simulink model "large_model"  (Simulink 8.0)
settings: Solver=ode45  StartTime=0.0  StopTime=10.0  RelTol=1e-3
(part 1 of 21)
settings Model: Creator=eng006  LastModifiedBy=rstarr
settings DataIOCC: SignalLoggingSaveFormat=ModelDataLogs
settings DebuggingCC: FcnCallInpInsideContextMsg=UseLocalSettings
settings GRTTargetCC: CombineOutputUpdateFcns=off  GRTInterface=on

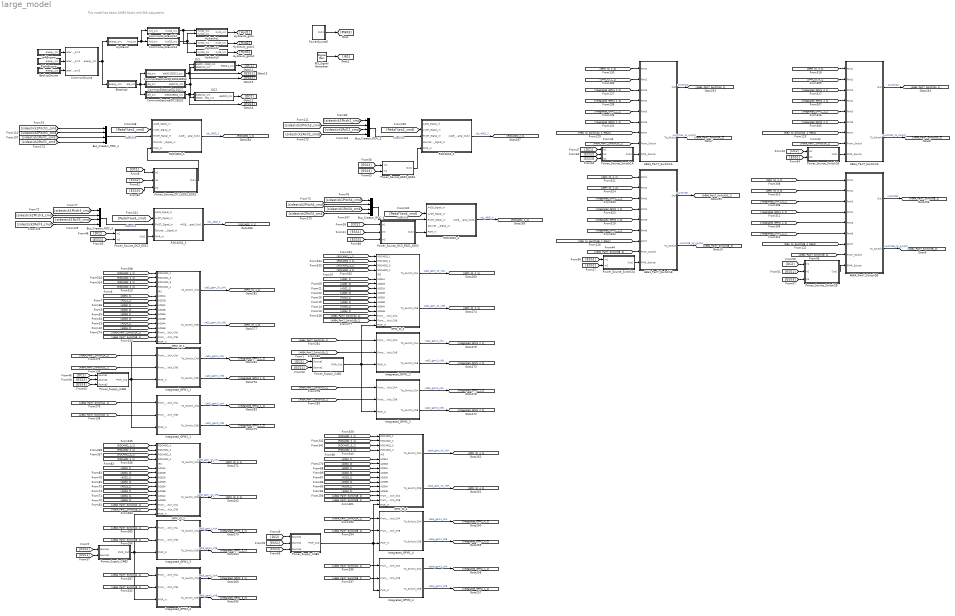
== system large_model ==
  note: This model has about 10959 blocks and 593 subsystems
  A664_Part7_Switch1A : Subsystem
  A664_Part7_Switch1B : Subsystem
  A664_Part7_Switch2A : Subsystem
  A664_Part7_Switch2B : Subsystem
  BackupSource : Subsystem
  Bus_Creator_RDC_{1..4} (4 alike) : BusCreator
  CommonElectricalDC1DC2 : Subsystem
  CommonElectricalDC1ESS1ESS3 : Subsystem
  CommonElectricalDC2ESS2 : Subsystem
  CommonHydraulic12 : Subsystem
  CommonHydraulic13 : Subsystem
  CommonSource : Subsystem
  DC1 : Subsystem
  DC2 : Subsystem
  Electrical : Subsystem
  GPM_IO_1 : Subsystem
  GPM_IO_2 : Subsystem
  GPM_IO_3 : Subsystem
  GPM_IO_4 : Subsystem
  Hydraulic : Subsystem
  Hydraulic1 : Subsystem
  Hydraulic2 : Subsystem
  Hydraulic3 : Subsystem
  IRS_Signal Generator : SignalGenerator
  Integrated_GPM1_1 : Subsystem
  Integrated_GPM1_2 : Subsystem
  Integrated_GPM1_3 : Subsystem
  Integrated_GPM1_4 : Subsystem
  Integrated_GPM2_1 : Subsystem
  Integrated_GPM2_2 : Subsystem
  Integrated_GPM2_3 : Subsystem
  Integrated_GPM2_4 : Subsystem
  LeftEngine : Subsystem
  PowerSource5 : Subsystem
  Power_Source_DC1_ESS2_ESS3 : Subsystem
  Power_Source_DC2_ESS1 : Subsystem
  Power_Source_DC2_ESS1_ESS3 : Subsystem
  Power_Source_ESS2_ESS3 : Subsystem
  Power_Source_Switch1A : Subsystem
  Power_Source_Switch1B : Subsystem
  Power_Source_Switch2A : Subsystem
  Power_Source_Switch2B : Subsystem
  Power_Supply_CAB1 : Subsystem
  Power_Supply_CAB2 : Subsystem
  Power_Supply_CAB3 : Subsystem
  Power_Supply_CAB4 : Subsystem
  RDC4002_1 : Subsystem
  RDC4002_2 : Subsystem
  RDC4002_3 : Subsystem
  RDC4002_4 : Subsystem
  RightEngine : Subsystem
  wires:
  Power_Supply_CAB1/1(PWR_Out) -> Integrated_GPM1_1/3(PWR_In), Integrated_GPM2_1/3(PWR_In), GPM_IO_1/16(PWR_In)
  A664_Part7_Switch2A/1(Out) -> [A664_Part7_Switch2A_1]  "switch2A"
  Power_Supply_CAB2/1(PWR_Out) -> GPM_IO_2/16(PWR_In), Integrated_GPM2_2/3(PWR_In), Integrated_GPM1_2/3(PWR_In)
  PowerSource5/1(Out1) -> [PWR3]
  IRS_Signal Generator/1 -> [IRS]
  Bus_Creator_RDC_1/1 -> RDC4002_1/3(RVDT_Signal_In)  "sidesticks"
  Bus_Creator_RDC_2/1 -> RDC4002_2/3(RVDT_Signal_In)  "sidesticks"
  Bus_Creator_RDC_3/1 -> RDC4002_3/3(RVDT_Signal_In)  "sidesticks"
  Bus_Creator_RDC_4/1 -> RDC4002_4/3(RVDT_Signal_In)  "sidesticks"
  Power_Supply_CAB3/1(PWR_Out) -> GPM_IO_3/16(PWR_In), Integrated_GPM1_3/3(PWR_In), Integrated_GPM2_3/3(PWR_In)
  A664_Part7_Switch1B/1(Out) -> [A664_Part7_Switch1B_1]  "switch1B"
  A664_Part7_Switch2B/1(Out) -> [A664_Part7_Switch2B_1]  "switch2B"
  Power_Supply_CAB4/1(PWR_Out) -> Integrated_GPM2_4/3(PWR_In), Integrated_GPM1_4/3(PWR_In), GPM_IO_4/16(PWR_In)
  [DC1] -> Power_Supply_CAB1/1(Source1)
  [DC2] -> Power_Supply_CAB2/1(Source1)
  [DC2] -> Power_Supply_CAB4/1(Source1)
  [ESS1] -> Power_Supply_CAB4/2(Source2)
  [ESS1] -> Power_Supply_CAB2/2(Source2)
  [ESS1] -> Power_Supply_CAB3/2(Source2)
  [ESS2] -> Power_Supply_CAB3/1(Source1)
  [ESS2] -> Power_Supply_CAB1/2(Source2)
  [DC2] -> Power_Source_DC2_ESS1_ESS3/1(In1)
  [ESS1] -> Power_Source_DC2_ESS1_ESS3/2(In2)
  [ESS3] -> Power_Source_DC2_ESS1_ESS3/3(In3)
  Power_Source_DC2_ESS1_ESS3/1(Out1) -> RDC4002_4/5(PWR_In)
  [DC2] -> Power_Source_DC2_ESS1/1(In1)
  [ESS1] -> Power_Source_DC2_ESS1/2(In2)
  Power_Source_DC2_ESS1/1(Out1) -> RDC4002_3/5(PWR_In)
  [ESS2] -> Power_Source_ESS2_ESS3/1(In1)
  [ESS3] -> Power_Source_ESS2_ESS3/2(In2)
  Power_Source_ESS2_ESS3/1(Out1) -> RDC4002_2/5(PWR_In)
  [DC1] -> Power_Source_DC1_ESS2_ESS3/1(In1)
  [ESS2] -> Power_Source_DC1_ESS2_ESS3/2(In2)
  [ESS3] -> Power_Source_DC1_ESS2_ESS3/3(In3)
  Power_Source_DC1_ESS2_ESS3/1(Out1) -> RDC4002_1/5(PWR_In)
  [DC2] -> Power_Source_Switch2B/1(In1)
  [ESS1] -> Power_Source_Switch2B/2(In2)
  [ESS3] -> Power_Source_Switch2B/3(In3)
  [ESS2] -> Power_Source_Switch2A/2(In2)
  [ESS1] -> Power_Source_Switch2A/1(In1)
  Power_Source_Switch2A/1(Out1) -> A664_Part7_Switch1B/9(PWR_Source)
  [DC2] -> Power_Source_Switch1B/1(In1)
  [ESS1] -> Power_Source_Switch1B/2(In2)
  Power_Source_Switch1B/1(Out1) -> A664_Part7_Switch2A/9(PWR_Source)
  [DC1] -> Power_Source_Switch1A/1(In1)
  [ESS2] -> Power_Source_Switch1A/2(In2)
  [ESS3] -> Power_Source_Switch1A/3(In3)
  Power_Source_Switch1A/1(Out1) -> A664_Part7_Switch1A/9(PWR_Source)
  [ESS3] -> Power_Supply_CAB4/3(Source3)
  [ESS3] -> Power_Supply_CAB1/3(Source3)
  [sidestick1Pitch1_cmd] -> Bus_Creator_RDC_1/1
  [sidestick1Pitch2_cmd] -> Bus_Creator_RDC_2/1
  [sidestick1Pitch3_cmd] -> Bus_Creator_RDC_3/1
  [sidestick1Pitch4_cmd] -> Bus_Creator_RDC_4/1
  [sidestick2Pitch1_cmd] -> Bus_Creator_RDC_1/2
  [PedalYaw1_cmd] -> RDC4002_1/2(LVDT_Signal_In)
  [sidestick2Pitch2_cmd] -> Bus_Creator_RDC_2/2
  [PedalYaw2_cmd] -> RDC4002_2/2(LVDT_Signal_In)
  [PedalYaw3_cmd] -> RDC4002_3/2(LVDT_Signal_In)
  [PedalYaw4_cmd] -> RDC4002_4/2(LVDT_Signal_In)
  [sidestick2Pitch3_cmd] -> Bus_Creator_RDC_3/2
  [sidestick2Pitch4_cmd] -> Bus_Creator_RDC_4/2
  [sidestick1Roll1_cmd] -> Bus_Creator_RDC_1/3
  [sidestick2Roll1_cmd] -> Bus_Creator_RDC_1/4
  [sidestick1Roll2_cmd] -> Bus_Creator_RDC_2/3
  [sidestick2Roll2_cmd] -> Bus_Creator_RDC_2/4
  [sidestick1Roll3_cmd] -> Bus_Creator_RDC_3/3
  [sidestick2Roll3_cmd] -> Bus_Creator_RDC_3/4
  [sidestick1Roll4_cmd] -> Bus_Creator_RDC_4/3
  [sidestick2Roll4_cmd] -> Bus_Creator_RDC_4/4
  [ADS2_2] -> GPM_IO_4/9(ADS2B)
  [ADS3_2] -> GPM_IO_4/11(ADS3B)
  [ADS4_2] -> GPM_IO_4/13(ADS4B)
  A664_Part7_Switch1A/2(To_Switch) -> [A664_Part7_Switch1A_2]  "switch1A_to_switch"
  [A664_Part7_Switch1A_2] -> A664_Part7_Switch2A/8(From_Switch)
  A664_Part7_Switch2A/2(To_Switch) -> [A664_Part7_Switch2A_2]  "switch2A_to_switch"
  [A664_Part7_Switch2A_2] -> A664_Part7_Switch1A/8(From_Switch)
  A664_Part7_Switch1A/1(Out) -> [A664_Part7_Switch1A_1]  "switch1A"
  A664_Part7_Switch2B/2(To_Switch) -> [A664_Part7_Switch2B_2]  "switch2B_to_switch"
  [A664_Part7_Switch2B_2] -> A664_Part7_Switch1B/8(From_Switch)
  A664_Part7_Switch1B/2(To_Switch) -> [A664_Part7_Switch1B_2]  "switch1B_to_switch"
  [A664_Part7_Switch1B_2] -> A664_Part7_Switch2B/8(From_Switch)
  [A664_Part7_Switch1A_1] -> GPM_IO_1/14(From_Switch_ChA)
  [A664_Part7_Switch1A_1] -> Integrated_GPM1_1/1(From_Switch_ChA)
  [A664_Part7_Switch1A_1] -> Integrated_GPM2_1/1(From_Switch_ChA)
  [A664_Part7_Switch1B_1] -> GPM_IO_2/15(From_Switch_ChB)
  [A664_Part7_Switch1B_1] -> Integrated_GPM2_1/2(From_Switch_ChB)
  [A664_Part7_Switch1B_1] -> Integrated_GPM1_1/2(From_Switch_ChB)
  [A664_Part7_Switch1B_1] -> GPM_IO_1/15(From_Switch_ChB)
  [A664_Part7_Switch1A_1] -> GPM_IO_2/14(From_Switch_ChA)
  [A664_Part7_Switch1A_1] -> Integrated_GPM1_2/1(From_Switch_ChA)
  [A664_Part7_Switch1B_1] -> Integrated_GPM2_2/2(From_Switch_ChB)
  [A664_Part7_Switch1B_1] -> Integrated_GPM1_2/2(From_Switch_ChB)
  [A664_Part7_Switch1A_1] -> Integrated_GPM2_2/1(From_Switch_ChA)
  [A664_Part7_Switch2A_1] -> Integrated_GPM1_3/1(From_Switch_ChA)
  [A664_Part7_Switch2A_1] -> Integrated_GPM2_3/1(From_Switch_ChA)
  [A664_Part7_Switch1B_1] -> GPM_IO_4/14(From_Switch_ChA)
  [A664_Part7_Switch2A_1] -> Integrated_GPM1_4/1(From_Switch_ChA)
  [A664_Part7_Switch2A_1] -> Integrated_GPM2_4/1(From_Switch_ChA)
  [A664_Part7_Switch2B_1] -> Integrated_GPM1_3/2(From_Switch_ChB)
  [A664_Part7_Switch2B_1] -> Integrated_GPM2_3/2(From_Switch_ChB)
  [A664_Part7_Switch2B_1] -> GPM_IO_4/15(From_Switch_ChB)
  [A664_Part7_Switch2B_1] -> Integrated_GPM1_4/2(From_Switch_ChB)
  [A664_Part7_Switch2B_1] -> Integrated_GPM2_4/2(From_Switch_ChB)
  Integrated_GPM2_4/2(To_Switch_ChB) -> [Integrated_GPM2_4_2]  "cab4_gpm2_chB"
  [Integrated_GPM2_4_2] -> A664_Part7_Switch2B/5(Port5)
  Integrated_GPM2_4/1(To_Switch_ChA) -> [Integrated_GPM2_4_1]  "cab4_gpm2_chA"
  Integrated_GPM1_4/2(To_Switch_ChB) -> [Integrated_GPM1_4_2]  "cab4_gpm1_chB"
  [Integrated_GPM1_4_2] -> A664_Part7_Switch2B/3(Port3)
  Integrated_GPM1_4/1(To_Switch_ChA) -> [Integrated_GPM1_4_1]  "cab4_gpm1_chA"
  GPM_IO_4/2(To_Switch_ChB) -> [GPM_IO_4_2]  "cab4_gpm_IO_chB"
  [GPM_IO_4_2] -> A664_Part7_Switch2B/1(Port1)
  GPM_IO_4/1(To_Switch_ChA) -> [GPM_IO_4_1]  "cab4_gpm_IO_chA"
  GPM_IO_3/2(To_Switch_ChB) -> [GPM_IO_3_2]  "cab3_gpm_IO_chB"
  [GPM_IO_3_2] -> A664_Part7_Switch2B/2(Port2)
  Integrated_GPM1_3/2(To_Switch_ChB) -> [Integrated_GPM1_3_2]  "cab3_gpm1_chB"
  [Integrated_GPM1_3_2] -> A664_Part7_Switch2B/4(Port4)
  Integrated_GPM2_3/2(To_Switch_ChB) -> [Integrated_GPM2_3_2]  "cab3_gpm2_chB"
  [Integrated_GPM2_3_2] -> A664_Part7_Switch2B/6(Port6)
  Integrated_GPM2_3/1(To_Switch_ChA) -> [Integrated_GPM2_3_1]  "cab3_gpm2_chA"
  Integrated_GPM1_3/1(To_Switch_ChA) -> [Integrated_GPM1_3_1]  "cab3_gpm1_chA"
  GPM_IO_3/1(To_Switch_ChA) -> [GPM_IO_3_1]  "cab3_gpm_IO_chA"
  Power_Source_Switch2B/1(Out1) -> A664_Part7_Switch2B/9(PWR_Source)
  Integrated_GPM2_2/2(To_Switch_ChB) -> [Integrated_GPM2_2_2]  "cab2_gpm2_chB"
  Integrated_GPM1_2/2(To_Switch_ChB) -> [Integrated_GPM1_2_2]  "cab2_gpm1_chB"
  GPM_IO_2/2(To_Switch_ChB) -> [GPM_IO_2_2]  "cab2_gpm_IO_chB"
  Integrated_GPM2_1/2(To_Switch_ChB) -> [Integrated_GPM2_1_2]  "cab1_gpm2_chB"
  Integrated_GPM1_1/2(To_Switch_ChB) -> [Integrated_GPM1_1_2]  "cab1_gpm1_chB"
  GPM_IO_1/2(To_Switch_ChB) -> [GPM_IO_1_2]  "cab1_gpm_IO_chB"
  Integrated_GPM2_2/1(To_Switch_ChA) -> [Integrated_GPM2_2_1]  "cab2_gpm2_chA"
  Integrated_GPM1_2/1(To_Switch_ChA) -> [Integrated_GPM1_2_1]  "cab2_gpm1_chA"
  [Integrated_GPM1_1_2] -> A664_Part7_Switch1A/4(Port4)
  GPM_IO_2/1(To_Switch_ChA) -> [GPM_IO_2_1]  "cab2_gpm_IO_chA"
  Integrated_GPM1_1/1(To_Switch_ChA) -> [Integrated_GPM1_1_1]  "cab1_gpm1_chA"
  [Integrated_GPM1_2_2] -> A664_Part7_Switch1A/3(Port3)
  GPM_IO_1/1(To_Switch_ChA) -> [GPM_IO_1_1]  "cab1_gpm_IO_chA"
  [GPM_IO_2_2] -> A664_Part7_Switch1A/1(Port1)
  Integrated_GPM2_1/1(To_Switch_ChA) -> [Integrated_GPM2_1_1]  "cab1_gpm2_chA"
  [ADS1_1] -> GPM_IO_1/6(ADS1A)
  [ADS1_1] -> GPM_IO_4/6(ADS1A)
  [ADS1_2] -> GPM_IO_1/7(ADS1B)
  [ADS1_2] -> GPM_IO_4/7(ADS1B)
  [ADS2_1] -> GPM_IO_1/8(ADS2A)
  [ADS2_1] -> GPM_IO_4/8(ADS2A)
  [ADS4_2] -> GPM_IO_1/13(ADS4B)
  [ADS4_1] -> GPM_IO_1/12(ADS4A)
  [ADS3_2] -> GPM_IO_1/11(ADS3B)
  [ADS3_1] -> GPM_IO_1/10(ADS3A)
  [ADS2_2] -> GPM_IO_1/9(ADS2B)
  [ADS1_1] -> GPM_IO_2/6(ADS1A)
  [ADS1_2] -> GPM_IO_2/7(ADS1B)
  [ADS2_1] -> GPM_IO_2/8(ADS2A)
  [ADS2_2] -> GPM_IO_2/9(ADS2B)
  [ADS3_1] -> GPM_IO_2/10(ADS3A)
  [ADS3_2] -> GPM_IO_2/11(ADS3B)
  [ADS4_1] -> GPM_IO_2/12(ADS4A)
  [ADS4_2] -> GPM_IO_2/13(ADS4B)
  [ADS1_1] -> GPM_IO_3/6(ADS1A)
  [ADS1_2] -> GPM_IO_3/7(ADS1B)
  [ADS2_1] -> GPM_IO_3/8(ADS2A)
  [ADS2_2] -> GPM_IO_3/9(ADS2B)
  [ADS3_1] -> GPM_IO_3/10(ADS3A)
  [ADS3_2] -> GPM_IO_3/11(ADS3B)
  [ADS4_1] -> GPM_IO_3/12(ADS4A)
  [ADS4_2] -> GPM_IO_3/13(ADS4B)
  [ADS3_1] -> GPM_IO_4/10(ADS3A)
  [ADS4_1] -> GPM_IO_4/12(ADS4A)
  [A664_Part7_Switch2A_1] -> GPM_IO_3/14(From_Switch_ChA)
  [A664_Part7_Switch2B_1] -> GPM_IO_3/15(From_Switch_ChB)
  RDC4002_1/1(A429_Signal_Out1) -> [RDC4002_1_1]  "rdc_4002_1"
  [RDC4002_1_1] -> GPM_IO_1/1(RDC4002_1)
  RDC4002_4/1(A429_Signal_Out1) -> [RDC4002_4_1]  "rdc_4002_4"
  [RDC4002_4_1] -> GPM_IO_1/4(RDC4002_4)
  RDC4002_3/1(A429_Signal_Out1) -> [RDC4002_3_1]  "rdc_4002_3"
  [RDC4002_3_1] -> GPM_IO_1/3(RDC4002_3)
  RDC4002_2/1(A429_Signal_Out1) -> [RDC4002_2_1]  "rdc_4002_2"
  [RDC4002_2_1] -> GPM_IO_1/2(RDC4002_2)
  [RDC4002_1_1] -> GPM_IO_2/1(RDC4002_1)
  [RDC4002_2_1] -> GPM_IO_2/2(RDC4002_2)
  [RDC4002_3_1] -> GPM_IO_2/3(RDC4002_3)
  [RDC4002_4_1] -> GPM_IO_2/4(RDC4002_4)
  [RDC4002_1_1] -> GPM_IO_3/1(RDC4002_1)
  [RDC4002_2_1] -> GPM_IO_3/2(RDC4002_2)
  [RDC4002_3_1] -> GPM_IO_3/3(RDC4002_3)
  [RDC4002_4_1] -> GPM_IO_3/4(RDC4002_4)
  [RDC4002_1_1] -> GPM_IO_4/1(RDC4002_1)
  [RDC4002_2_1] -> GPM_IO_4/2(RDC4002_2)
  [RDC4002_3_1] -> GPM_IO_4/3(RDC4002_3)
  [RDC4002_4_1] -> GPM_IO_4/4(RDC4002_4)
  [GPM_IO_2_2] -> A664_Part7_Switch1B/1(Port1)
  [GPM_IO_1_2] -> A664_Part7_Switch1B/2(Port2)
  [Integrated_GPM1_2_2] -> A664_Part7_Switch1B/3(Port3)
  [Integrated_GPM1_1_2] -> A664_Part7_Switch1B/4(Port4)
  [Integrated_GPM2_2_2] -> A664_Part7_Switch1B/5(Port5)
  [Integrated_GPM2_1_2] -> A664_Part7_Switch1B/6(Port6)
  [GPM_IO_3_1] -> A664_Part7_Switch2A/1(Port1)
  [GPM_IO_4_1] -> A664_Part7_Switch2A/2(Port2)
  [Integrated_GPM1_3_1] -> A664_Part7_Switch2A/3(Port3)
  [Integrated_GPM1_4_1] -> A664_Part7_Switch2A/4(Port4)
  [Integrated_GPM2_3_1] -> A664_Part7_Switch2A/5(Port5)
  [Integrated_GPM2_4_1] -> A664_Part7_Switch2A/6(Port6)
  [GPM_IO_1_2] -> A664_Part7_Switch1A/2(Port2)
  [Integrated_GPM2_2_2] -> A664_Part7_Switch1A/5(Port5)
  [Integrated_GPM2_1_2] -> A664_Part7_Switch1A/6(Port6)
  CommonSource/1(energy_src) -> Electrical/1(energy_src), Hydraulic/1(energy_src)
  BackupSource/1(energy_src) -> CommonSource/3(energy_src3)
  RightEngine/1(energy_src) -> CommonSource/2(energy_src2)
  LeftEngine/1(energy_src) -> CommonSource/1(energy_src1)
  CommonHydraulic13/1(hyd13_src) -> Hydraulic3/1(hyd13_src), Hydraulic1/2(hyd13_src)
  CommonHydraulic12/1(hyd12_src) -> Hydraulic2/1(hyd12_src), Hydraulic1/1(hyd12_src)
  Hydraulic/1(hyd_src) -> CommonHydraulic12/1(hyd_src), CommonHydraulic13/1(hyd_src)
  CommonElectricalDC1DC2/1(eleDC12_src) -> DC1/2(eleDC12_src), DC2/2(eleDC2ESS2_src)
  DC2/1(eleDC2_src) -> [DC2]
  DC1/1(eleDC1_src) -> [DC1]
  CommonElectricalDC2ESS2/1(eleDC2ESS2_src) -> [ESS3], DC2/1(eleDC12_src)
  CommonElectricalDC1ESS1ESS3/1(eleDC1ESS13_src) -> [ESS2], [ESS1], DC1/1(eleDC1ESS13_src)
  Electrical/1(ele_src) -> CommonElectricalDC1ESS1ESS3/1(ele_src), CommonElectricalDC2ESS2/1(ele_src), CommonElectricalDC1DC2/1(ele_src)
  Hydraulic1/1(hyd1_src) -> [Hyd1]
  Hydraulic2/1(hyd2_src) -> [Hyd2]
  Hydraulic3/1(hyd3_src) -> [Hyd3]
  [REU_to_Switch2B_1_REUs] -> A664_Part7_Switch2B/7(Port7)
  [REU_to_Switch2A_1_REUs] -> A664_Part7_Switch2A/7(Port7)
  [REU_to_Switch1B_1_REUs] -> A664_Part7_Switch1B/7(Port7)
  [REU_to_Switch1A_1_REUs] -> A664_Part7_Switch1A/7(Port7)

== system large_model/A664_Part7_Switch1A ==
  note: Atenção! O sinal de From_Switch não deve ser propagado para a saída To_Switch.
      | Isto é feito para evitar loops entre switches.
  Port1 : Inport
  Port2 : Inport  Port=2
  Port3 : Inport  Port=3
  Port4 : Inport  Port=4
  Port5 : Inport  Port=5
  Port6 : Inport  Port=6
  Port7 : Inport  Port=7
  From_Switch : Inport  Port=8
  PWR_Source : Inport  Port=9
  Bus Direct : BusCreator  Inputs=8
  Bus to Switch : BusCreator  Inputs=7
  Switch Hardware : Subsystem
  Out : Outport
  To_Switch : Outport  Port=2
  wires:
  Bus Direct/1 -> Switch Hardware/1(In1)
  PWR_Source/1 -> Switch Hardware/3(PWR)
  Switch Hardware/1(Out1) -> Out/1
  Switch Hardware/2(Out2) -> To_Switch/1
  Bus to Switch/1 -> Switch Hardware/2(In2)
  Port1/1 -> Bus Direct/1, Bus to Switch/1
  Port2/1 -> Bus Direct/2, Bus to Switch/2
  Port3/1 -> Bus Direct/3, Bus to Switch/3
  Port4/1 -> Bus Direct/4, Bus to Switch/4
  Port5/1 -> Bus Direct/5, Bus to Switch/5
  Port6/1 -> Bus Direct/6, Bus to Switch/6
  Port7/1 -> Bus Direct/7, Bus to Switch/7
  From_Switch/1 -> Bus Direct/8

== system large_model/A664_Part7_Switch1B ==
  note: Atenção! O sinal de From_Switch não deve ser propagado para a saída To_Switch.
      | Isto é feito para evitar loops entre switches.
  Port1 : Inport
  Port2 : Inport  Port=2
  Port3 : Inport  Port=3
  Port4 : Inport  Port=4
  Port5 : Inport  Port=5
  Port6 : Inport  Port=6
  Port7 : Inport  Port=7
  From_Switch : Inport  Port=8
  PWR_Source : Inport  Port=9
  Bus Direct : BusCreator  Inputs=8
  Bus to Switch : BusCreator  Inputs=7
  Switch Hardware : Subsystem
  Out : Outport
  To_Switch : Outport  Port=2
  wires:
  Bus Direct/1 -> Switch Hardware/1(In1)
  PWR_Source/1 -> Switch Hardware/3(PWR)
  Switch Hardware/1(Out1) -> Out/1
  Switch Hardware/2(Out2) -> To_Switch/1
  Bus to Switch/1 -> Switch Hardware/2(In2)
  Port1/1 -> Bus Direct/1, Bus to Switch/1
  Port2/1 -> Bus Direct/2, Bus to Switch/2
  Port3/1 -> Bus Direct/3, Bus to Switch/3
  Port4/1 -> Bus Direct/4, Bus to Switch/4
  Port5/1 -> Bus Direct/5, Bus to Switch/5
  Port6/1 -> Bus Direct/6, Bus to Switch/6
  Port7/1 -> Bus Direct/7, Bus to Switch/7
  From_Switch/1 -> Bus Direct/8

== system large_model/A664_Part7_Switch2A ==
  note: Atenção! O sinal de From_Switch não deve ser propagado para a saída To_Switch.
      | Isto é feito para evitar loops entre switches.
  Port1 : Inport
  Port2 : Inport  Port=2
  Port3 : Inport  Port=3
  Port4 : Inport  Port=4
  Port5 : Inport  Port=5
  Port6 : Inport  Port=6
  Port7 : Inport  Port=7
  From_Switch : Inport  Port=8
  PWR_Source : Inport  Port=9
  Bus Direct : BusCreator  Inputs=8
  Bus to Switch : BusCreator  Inputs=7
  Switch Hardware : Subsystem
  Out : Outport
  To_Switch : Outport  Port=2
  wires:
  Bus Direct/1 -> Switch Hardware/1(In1)
  PWR_Source/1 -> Switch Hardware/3(PWR)
  Switch Hardware/1(Out1) -> Out/1
  Switch Hardware/2(Out2) -> To_Switch/1
  Bus to Switch/1 -> Switch Hardware/2(In2)
  Port1/1 -> Bus Direct/1, Bus to Switch/1
  Port2/1 -> Bus Direct/2, Bus to Switch/2
  Port3/1 -> Bus Direct/3, Bus to Switch/3
  Port4/1 -> Bus Direct/4, Bus to Switch/4
  Port5/1 -> Bus Direct/5, Bus to Switch/5
  Port6/1 -> Bus Direct/6, Bus to Switch/6
  Port7/1 -> Bus Direct/7, Bus to Switch/7
  From_Switch/1 -> Bus Direct/8

== system large_model/A664_Part7_Switch2B ==
  note: Atenção! O sinal de From_Switch não deve ser propagado para a saída To_Switch.
      | Isto é feito para evitar loops entre switches.
  Port1 : Inport
  Port2 : Inport  Port=2
  Port3 : Inport  Port=3
  Port4 : Inport  Port=4
  Port5 : Inport  Port=5
  Port6 : Inport  Port=6
  Port7 : Inport  Port=7
  From_Switch : Inport  Port=8
  PWR_Source : Inport  Port=9
  Bus Direct : BusCreator  Inputs=8
  Bus to Switch : BusCreator  Inputs=7
  Switch Hardware : Subsystem
  Out : Outport
  To_Switch : Outport  Port=2
  wires:
  Bus Direct/1 -> Switch Hardware/1(In1)
  PWR_Source/1 -> Switch Hardware/3(PWR)
  Switch Hardware/1(Out1) -> Out/1
  Switch Hardware/2(Out2) -> To_Switch/1
  Bus to Switch/1 -> Switch Hardware/2(In2)
  Port1/1 -> Bus Direct/1, Bus to Switch/1
  Port2/1 -> Bus Direct/2, Bus to Switch/2
  Port3/1 -> Bus Direct/3, Bus to Switch/3
  Port4/1 -> Bus Direct/4, Bus to Switch/4
  Port5/1 -> Bus Direct/5, Bus to Switch/5
  Port6/1 -> Bus Direct/6, Bus to Switch/6
  Port7/1 -> Bus Direct/7, Bus to Switch/7
  From_Switch/1 -> Bus Direct/8

== system large_model/BackupSource ==
  energy_src : Outport

== system large_model/CommonElectricalDC1DC2 ==
  ele_src : Inport
  eleDC12_src : Outport

== system large_model/CommonElectricalDC1ESS1ESS3 ==
  ele_src : Inport
  eleDC1ESS13_src : Outport

== system large_model/CommonElectricalDC2ESS2 ==
  ele_src : Inport
  eleDC2ESS2_src : Outport

== system large_model/CommonHydraulic12 ==
  hyd_src : Inport
  hyd12_src : Outport

== system large_model/CommonHydraulic13 ==
  hyd_src : Inport
  hyd13_src : Outport

== system large_model/CommonSource ==
  energy_src1 : Inport
  energy_src2 : Inport  Port=2
  energy_src3 : Inport  Port=3
  energy_src : Outport

== system large_model/DC1 ==
  eleDC1ESS13_src : Inport
  eleDC12_src : Inport  Port=2
  eleDC1_src : Outport

== system large_model/DC2 ==
  eleDC12_src : Inport
  eleDC2ESS2_src : Inport  Port=2
  eleDC2_src : Outport

== system large_model/Electrical ==
  energy_src : Inport
  ele_src : Outport

== system large_model/GPM_IO_1 ==
  RDC4002_1 : Inport
  RDC4002_2 : Inport  Port=2
  RDC4002_3 : Inport  Port=3
  RDC4002_4 : Inport  Port=4
  IRS : Inport  Port=5
  ADS1A : Inport  Port=6
  ADS1B : Inport  Port=7
  ADS2A : Inport  Port=8
  ADS2B : Inport  Port=9
  ADS3A : Inport  Port=10
  ADS3B : Inport  Port=11
  ADS4A : Inport  Port=12
  ADS4B : Inport  Port=13
  From_Switch_ChA : Inport  Port=14
  From_Switch_ChB : Inport  Port=15
  PWR_In : Inport  Port=16
  IO_Card : Subsystem
  Integrated_GPM0 : Subsystem
  To_Switch_ChA : Outport
  To_Switch_ChB : Outport  Port=2
  wires:
  IO_Card/1(A429_Out1) -> Integrated_GPM0/1(From_IO_Card)
  PWR_In/1 -> IO_Card/14(PWR_In), Integrated_GPM0/2(PWR_In)
  RDC4002_1/1 -> IO_Card/1(RDC4002_1)
  RDC4002_2/1 -> IO_Card/2(RDC4002_2)
  Integrated_GPM0/1(To_Switch_ChA) -> To_Switch_ChA/1
  RDC4002_3/1 -> IO_Card/3(RDC4002_3)
  RDC4002_4/1 -> IO_Card/4(RDC4002_4)
  From_Switch_ChA/1 -> Integrated_GPM0/3(From_Switch_ChA)
  Integrated_GPM0/2(To_Switch_ChB) -> To_Switch_ChB/1
  IRS/1 -> IO_Card/5(IRS)
  From_Switch_ChB/1 -> Integrated_GPM0/4(From_Switch_ChB)
  ADS1A/1 -> IO_Card/6(ADS1)
  ADS1B/1 -> IO_Card/7(ADS2)
  ADS2A/1 -> IO_Card/8(ADS3)
  ADS2B/1 -> IO_Card/9(ADS4)
  ADS3A/1 -> IO_Card/10(ADS5)
  ADS3B/1 -> IO_Card/11(ADS6)
  ADS4A/1 -> IO_Card/12(ADS7)
  ADS4B/1 -> IO_Card/13(ADS8)

== system large_model/GPM_IO_2 ==
  RDC4002_1 : Inport
  RDC4002_2 : Inport  Port=2
  RDC4002_3 : Inport  Port=3
  RDC4002_4 : Inport  Port=4
  IRS : Inport  Port=5
  ADS1A : Inport  Port=6
  ADS1B : Inport  Port=7
  ADS2A : Inport  Port=8
  ADS2B : Inport  Port=9
  ADS3A : Inport  Port=10
  ADS3B : Inport  Port=11
  ADS4A : Inport  Port=12
  ADS4B : Inport  Port=13
  From_Switch_ChA : Inport  Port=14
  From_Switch_ChB : Inport  Port=15
  PWR_In : Inport  Port=16
  IO_Card : Subsystem
  Integrated_GPM0 : Subsystem
  To_Switch_ChA : Outport
  To_Switch_ChB : Outport  Port=2
  wires:
  IO_Card/1(A429_Out1) -> Integrated_GPM0/1(From_IO_Card)
  PWR_In/1 -> IO_Card/14(PWR_In), Integrated_GPM0/2(PWR_In)
  RDC4002_1/1 -> IO_Card/1(RDC4002_1)
  RDC4002_2/1 -> IO_Card/2(RDC4002_2)
  Integrated_GPM0/1(To_Switch_ChA) -> To_Switch_ChA/1
  RDC4002_3/1 -> IO_Card/3(RDC4002_3)
  RDC4002_4/1 -> IO_Card/4(RDC4002_4)
  From_Switch_ChA/1 -> Integrated_GPM0/3(From_Switch_ChA)
  Integrated_GPM0/2(To_Switch_ChB) -> To_Switch_ChB/1
  IRS/1 -> IO_Card/5(IRS)
  From_Switch_ChB/1 -> Integrated_GPM0/4(From_Switch_ChB)
  ADS1A/1 -> IO_Card/6(ADS1)
  ADS1B/1 -> IO_Card/7(ADS2)
  ADS2A/1 -> IO_Card/8(ADS3)
  ADS2B/1 -> IO_Card/9(ADS4)
  ADS3A/1 -> IO_Card/10(ADS5)
  ADS3B/1 -> IO_Card/11(ADS6)
  ADS4A/1 -> IO_Card/12(ADS7)
  ADS4B/1 -> IO_Card/13(ADS8)

== system large_model/GPM_IO_3 ==
  RDC4002_1 : Inport
  RDC4002_2 : Inport  Port=2
  RDC4002_3 : Inport  Port=3
  RDC4002_4 : Inport  Port=4
  IRS : Inport  Port=5
  ADS1A : Inport  Port=6
  ADS1B : Inport  Port=7
  ADS2A : Inport  Port=8
  ADS2B : Inport  Port=9
  ADS3A : Inport  Port=10
  ADS3B : Inport  Port=11
  ADS4A : Inport  Port=12
  ADS4B : Inport  Port=13
  From_Switch_ChA : Inport  Port=14
  From_Switch_ChB : Inport  Port=15
  PWR_In : Inport  Port=16
  IO_Card : Subsystem
  Integrated_GPM0 : Subsystem
  To_Switch_ChA : Outport
  To_Switch_ChB : Outport  Port=2
  wires:
  IO_Card/1(A429_Out1) -> Integrated_GPM0/1(From_IO_Card)
  PWR_In/1 -> IO_Card/14(PWR_In), Integrated_GPM0/2(PWR_In)
  RDC4002_1/1 -> IO_Card/1(RDC4002_1)
  RDC4002_2/1 -> IO_Card/2(RDC4002_2)
  Integrated_GPM0/1(To_Switch_ChA) -> To_Switch_ChA/1
  RDC4002_3/1 -> IO_Card/3(RDC4002_3)
  RDC4002_4/1 -> IO_Card/4(RDC4002_4)
  From_Switch_ChA/1 -> Integrated_GPM0/3(From_Switch_ChA)
  Integrated_GPM0/2(To_Switch_ChB) -> To_Switch_ChB/1
  IRS/1 -> IO_Card/5(IRS)
  From_Switch_ChB/1 -> Integrated_GPM0/4(From_Switch_ChB)
  ADS1A/1 -> IO_Card/6(ADS1)
  ADS1B/1 -> IO_Card/7(ADS2)
  ADS2A/1 -> IO_Card/8(ADS3)
  ADS2B/1 -> IO_Card/9(ADS4)
  ADS3A/1 -> IO_Card/10(ADS5)
  ADS3B/1 -> IO_Card/11(ADS6)
  ADS4A/1 -> IO_Card/12(ADS7)
  ADS4B/1 -> IO_Card/13(ADS8)

== system large_model/GPM_IO_4 ==
  RDC4002_1 : Inport
  RDC4002_2 : Inport  Port=2
  RDC4002_3 : Inport  Port=3
  RDC4002_4 : Inport  Port=4
  IRS : Inport  Port=5
  ADS1A : Inport  Port=6
  ADS1B : Inport  Port=7
  ADS2A : Inport  Port=8
  ADS2B : Inport  Port=9
  ADS3A : Inport  Port=10
  ADS3B : Inport  Port=11
  ADS4A : Inport  Port=12
  ADS4B : Inport  Port=13
  From_Switch_ChA : Inport  Port=14
  From_Switch_ChB : Inport  Port=15
  PWR_In : Inport  Port=16
  IO_Card : Subsystem
  Integrated_GPM0 : Subsystem
  To_Switch_ChA : Outport
  To_Switch_ChB : Outport  Port=2
  wires:
  IO_Card/1(A429_Out1) -> Integrated_GPM0/1(From_IO_Card)
  PWR_In/1 -> IO_Card/14(PWR_In), Integrated_GPM0/2(PWR_In)
  RDC4002_1/1 -> IO_Card/1(RDC4002_1)
  RDC4002_2/1 -> IO_Card/2(RDC4002_2)
  Integrated_GPM0/1(To_Switch_ChA) -> To_Switch_ChA/1
  RDC4002_3/1 -> IO_Card/3(RDC4002_3)
  RDC4002_4/1 -> IO_Card/4(RDC4002_4)
  From_Switch_ChA/1 -> Integrated_GPM0/3(From_Switch_ChA)
  Integrated_GPM0/2(To_Switch_ChB) -> To_Switch_ChB/1
  IRS/1 -> IO_Card/5(IRS)
  From_Switch_ChB/1 -> Integrated_GPM0/4(From_Switch_ChB)
  ADS1A/1 -> IO_Card/6(ADS1)
  ADS1B/1 -> IO_Card/7(ADS2)
  ADS2A/1 -> IO_Card/8(ADS3)
  ADS2B/1 -> IO_Card/9(ADS4)
  ADS3A/1 -> IO_Card/10(ADS5)
  ADS3B/1 -> IO_Card/11(ADS6)
  ADS4A/1 -> IO_Card/12(ADS7)
  ADS4B/1 -> IO_Card/13(ADS8)

== system large_model/Hydraulic ==
  energy_src : Inport
  hyd_src : Outport

== system large_model/Hydraulic1 ==
  hyd12_src : Inport
  hyd13_src : Inport  Port=2
  hyd1_src : Outport

== system large_model/Hydraulic2 ==
  hyd12_src : Inport
  hyd2_src : Outport

== system large_model/Hydraulic3 ==
  hyd13_src : Inport
  hyd3_src : Outport

== system large_model/Integrated_GPM1_1 ==
  From_Switch_ChA : Inport
  From_Switch_ChB : Inport  Port=2
  PWR_In : Inport  Port=3
  End_System : Subsystem
  GPM : Subsystem
  To_Switch_ChA : Outport
  To_Switch_ChB : Outport  Port=2
  wires:
  GPM/1(To_ES) -> End_System/1(From_GPM)
  GPM/2(PWR_Out) -> End_System/4(PWR)
  PWR_In/1 -> GPM/2(PWR_In)
  From_Switch_ChA/1 -> End_System/2(From_Switch_ChA)
  End_System/2(To_Switch_ChA) -> To_Switch_ChA/1
  From_Switch_ChB/1 -> End_System/3(From_Switch_ChB)
  End_System/3(To_Switch_ChB) -> To_Switch_ChB/1
  End_System/1(To_GPM) -> GPM/1(From_ES)

== system large_model/Integrated_GPM1_2 ==
  From_Switch_ChA : Inport
  From_Switch_ChB : Inport  Port=2
  PWR_In : Inport  Port=3
  End_System : Subsystem
  GPM : Subsystem
  To_Switch_ChA : Outport
  To_Switch_ChB : Outport  Port=2
  wires:
  End_System/1(To_GPM) -> GPM/1(From_ES)
  End_System/3(To_Switch_ChB) -> To_Switch_ChB/1
  From_Switch_ChB/1 -> End_System/3(From_Switch_ChB)
  End_System/2(To_Switch_ChA) -> To_Switch_ChA/1
  From_Switch_ChA/1 -> End_System/2(From_Switch_ChA)
  PWR_In/1 -> GPM/2(PWR_In)
  GPM/2(PWR_Out) -> End_System/4(PWR)
  GPM/1(To_ES) -> End_System/1(From_GPM)

== system large_model/Integrated_GPM1_3 ==
  From_Switch_ChA : Inport
  From_Switch_ChB : Inport  Port=2
  PWR_In : Inport  Port=3
  End_System : Subsystem
  GPM : Subsystem
  To_Switch_ChA : Outport
  To_Switch_ChB : Outport  Port=2
  wires:
  End_System/1(To_GPM) -> GPM/1(From_ES)
  End_System/3(To_Switch_ChB) -> To_Switch_ChB/1
  From_Switch_ChB/1 -> End_System/3(From_Switch_ChB)
  End_System/2(To_Switch_ChA) -> To_Switch_ChA/1
  From_Switch_ChA/1 -> End_System/2(From_Switch_ChA)
  PWR_In/1 -> GPM/2(PWR_In)
  GPM/2(PWR_Out) -> End_System/4(PWR)
  GPM/1(To_ES) -> End_System/1(From_GPM)

== system large_model/Integrated_GPM1_4 ==
  From_Switch_ChA : Inport
  From_Switch_ChB : Inport  Port=2
  PWR_In : Inport  Port=3
  End_System : Subsystem
  GPM : Subsystem
  To_Switch_ChA : Outport
  To_Switch_ChB : Outport  Port=2
  wires:
  End_System/1(To_GPM) -> GPM/1(From_ES)
  End_System/3(To_Switch_ChB) -> To_Switch_ChB/1
  From_Switch_ChB/1 -> End_System/3(From_Switch_ChB)
  End_System/2(To_Switch_ChA) -> To_Switch_ChA/1
  From_Switch_ChA/1 -> End_System/2(From_Switch_ChA)
  PWR_In/1 -> GPM/2(PWR_In)
  GPM/2(PWR_Out) -> End_System/4(PWR)
  GPM/1(To_ES) -> End_System/1(From_GPM)

== system large_model/Integrated_GPM2_1 ==
  From_Switch_ChA : Inport
  From_Switch_ChB : Inport  Port=2
  PWR_In : Inport  Port=3
  End_System : Subsystem
  GPM : Subsystem
  To_Switch_ChA : Outport
  To_Switch_ChB : Outport  Port=2
  wires:
  End_System/1(To_GPM) -> GPM/1(From_ES)
  End_System/3(To_Switch_ChB) -> To_Switch_ChB/1
  From_Switch_ChB/1 -> End_System/3(From_Switch_ChB)
  End_System/2(To_Switch_ChA) -> To_Switch_ChA/1
  From_Switch_ChA/1 -> End_System/2(From_Switch_ChA)
  PWR_In/1 -> GPM/2(PWR_In)
  GPM/2(PWR_Out) -> End_System/4(PWR)
  GPM/1(To_ES) -> End_System/1(From_GPM)

== system large_model/Integrated_GPM2_2 ==
  From_Switch_ChA : Inport
  From_Switch_ChB : Inport  Port=2
  PWR_In : Inport  Port=3
  End_System : Subsystem
  GPM : Subsystem
  To_Switch_ChA : Outport
  To_Switch_ChB : Outport  Port=2
  wires:
  GPM/1(To_ES) -> End_System/1(From_GPM)
  GPM/2(PWR_Out) -> End_System/4(PWR)
  PWR_In/1 -> GPM/2(PWR_In)
  From_Switch_ChA/1 -> End_System/2(From_Switch_ChA)
  End_System/2(To_Switch_ChA) -> To_Switch_ChA/1
  From_Switch_ChB/1 -> End_System/3(From_Switch_ChB)
  End_System/3(To_Switch_ChB) -> To_Switch_ChB/1
  End_System/1(To_GPM) -> GPM/1(From_ES)

== system large_model/Integrated_GPM2_3 ==
  From_Switch_ChA : Inport
  From_Switch_ChB : Inport  Port=2
  PWR_In : Inport  Port=3
  End_System : Subsystem
  GPM : Subsystem
  To_Switch_ChA : Outport
  To_Switch_ChB : Outport  Port=2
  wires:
  GPM/1(To_ES) -> End_System/1(From_GPM)
  GPM/2(PWR_Out) -> End_System/4(PWR)
  PWR_In/1 -> GPM/2(PWR_In)
  From_Switch_ChA/1 -> End_System/2(From_Switch_ChA)
  End_System/2(To_Switch_ChA) -> To_Switch_ChA/1
  From_Switch_ChB/1 -> End_System/3(From_Switch_ChB)
  End_System/3(To_Switch_ChB) -> To_Switch_ChB/1
  End_System/1(To_GPM) -> GPM/1(From_ES)

== system large_model/Integrated_GPM2_4 ==
  From_Switch_ChA : Inport
  From_Switch_ChB : Inport  Port=2
  PWR_In : Inport  Port=3
  End_System : Subsystem
  GPM : Subsystem
  To_Switch_ChA : Outport
  To_Switch_ChB : Outport  Port=2
  wires:
  GPM/1(To_ES) -> End_System/1(From_GPM)
  GPM/2(PWR_Out) -> End_System/4(PWR)
  PWR_In/1 -> GPM/2(PWR_In)
  From_Switch_ChA/1 -> End_System/2(From_Switch_ChA)
  End_System/2(To_Switch_ChA) -> To_Switch_ChA/1
  From_Switch_ChB/1 -> End_System/3(From_Switch_ChB)
  End_System/3(To_Switch_ChB) -> To_Switch_ChB/1
  End_System/1(To_GPM) -> GPM/1(From_ES)

== system large_model/LeftEngine ==
  energy_src : Outport

== system large_model/PowerSource5 ==
  Out1 : Outport

== system large_model/Power_Source_DC1_ESS2_ESS3 ==
  In1 : Inport
  In2 : Inport  Port=2
  In3 : Inport  Port=3
  Out1 : Outport
  wires:
  In1/1 -> Out1/1

== system large_model/Power_Source_DC2_ESS1 ==
  In1 : Inport
  In2 : Inport  Port=2
  Out1 : Outport
  wires:
  In1/1 -> Out1/1

== system large_model/Power_Source_DC2_ESS1_ESS3 ==
  In1 : Inport
  In2 : Inport  Port=2
  In3 : Inport  Port=3
  Out1 : Outport
  wires:
  In1/1 -> Out1/1

== system large_model/Power_Source_ESS2_ESS3 ==
  In1 : Inport
  In2 : Inport  Port=2
  Out1 : Outport
  wires:
  In1/1 -> Out1/1

== system large_model/Power_Source_Switch1A ==
  In1 : Inport
  In2 : Inport  Port=2
  In3 : Inport  Port=3
  Out1 : Outport
  wires:
  In1/1 -> Out1/1

== system large_model/Power_Source_Switch1B ==
  In1 : Inport
  In2 : Inport  Port=2
  Out1 : Outport
  wires:
  In1/1 -> Out1/1

== system large_model/Power_Source_Switch2A ==
  In1 : Inport
  In2 : Inport  Port=2
  Out1 : Outport
  wires:
  In1/1 -> Out1/1

== system large_model/Power_Source_Switch2B ==
  In1 : Inport
  In2 : Inport  Port=2
  In3 : Inport  Port=3
  Out1 : Outport
  wires:
  In1/1 -> Out1/1

== system large_model/Power_Supply_CAB1 ==
  Source1 : Inport
  Source2 : Inport  Port=2
  Source3 : Inport  Port=3
  PWR_Out : Outport
  wires:
  Source1/1 -> PWR_Out/1

== system large_model/Power_Supply_CAB2 ==
  Source1 : Inport
  Source2 : Inport  Port=2
  PWR_Out : Outport
  wires:
  Source1/1 -> PWR_Out/1

== system large_model/Power_Supply_CAB3 ==
  Source1 : Inport
  Source2 : Inport  Port=2
  PWR_Out : Outport
  wires:
  Source1/1 -> PWR_Out/1

== system large_model/Power_Supply_CAB4 ==
  Source1 : Inport
  Source2 : Inport  Port=2
  Source3 : Inport  Port=3
  PWR_Out : Outport
  wires:
  Source1/1 -> PWR_Out/1

== system large_model/RDC4002_1 ==
  A429_Signal_In : Inport
  LVDT_Signal_In : Inport  Port=2
  RVDT_Signal_In : Inport  Port=3
  Discrete_Signal_In : Inport  Port=4
  PWR_In : Inport  Port=5
  Bus Creator : BusCreator
  RDC Hardware : Subsystem
  A429_Signal_Out1 : Outport
  wires:
  A429_Signal_In/1 -> Bus Creator/1
  LVDT_Signal_In/1 -> Bus Creator/2
  RVDT_Signal_In/1 -> Bus Creator/3
  Discrete_Signal_In/1 -> Bus Creator/4
  Bus Creator/1 -> RDC Hardware/1(In)
  RDC Hardware/1(Out) -> A429_Signal_Out1/1
  PWR_In/1 -> RDC Hardware/2(PWR_In)

== system large_model/RDC4002_2 ==
  A429_Signal_In : Inport
  LVDT_Signal_In : Inport  Port=2
  RVDT_Signal_In : Inport  Port=3
  Discrete_Signal_In : Inport  Port=4
  PWR_In : Inport  Port=5
  Bus Creator : BusCreator
  RDC Hardware : Subsystem
  A429_Signal_Out1 : Outport
  wires:
  A429_Signal_In/1 -> Bus Creator/1
  LVDT_Signal_In/1 -> Bus Creator/2
  RVDT_Signal_In/1 -> Bus Creator/3
  Discrete_Signal_In/1 -> Bus Creator/4
  Bus Creator/1 -> RDC Hardware/1(In)
  RDC Hardware/1(Out) -> A429_Signal_Out1/1
  PWR_In/1 -> RDC Hardware/2(PWR_In)

== system large_model/RDC4002_3 ==
  A429_Signal_In : Inport
  LVDT_Signal_In : Inport  Port=2
  RVDT_Signal_In : Inport  Port=3
  Discrete_Signal_In : Inport  Port=4
  PWR_In : Inport  Port=5
  Bus Creator : BusCreator
  RDC Hardware : Subsystem
  A429_Signal_Out1 : Outport
  wires:
  PWR_In/1 -> RDC Hardware/2(PWR_In)
  RDC Hardware/1(Out) -> A429_Signal_Out1/1
  Bus Creator/1 -> RDC Hardware/1(In)
  Discrete_Signal_In/1 -> Bus Creator/4
  RVDT_Signal_In/1 -> Bus Creator/3
  LVDT_Signal_In/1 -> Bus Creator/2
  A429_Signal_In/1 -> Bus Creator/1

== system large_model/RDC4002_4 ==
  A429_Signal_In : Inport
  LVDT_Signal_In : Inport  Port=2
  RVDT_Signal_In : Inport  Port=3
  Discrete_Signal_In : Inport  Port=4
  PWR_In : Inport  Port=5
  Bus Creator : BusCreator
  RDC Hardware : Subsystem
  A429_Signal_Out1 : Outport
  wires:
  A429_Signal_In/1 -> Bus Creator/1
  LVDT_Signal_In/1 -> Bus Creator/2
  RVDT_Signal_In/1 -> Bus Creator/3
  Discrete_Signal_In/1 -> Bus Creator/4
  Bus Creator/1 -> RDC Hardware/1(In)
  RDC Hardware/1(Out) -> A429_Signal_Out1/1
  PWR_In/1 -> RDC Hardware/2(PWR_In)

== system large_model/RightEngine ==
  energy_src : Outport

== system large_model/A664_Part7_Switch1A/Switch Hardware ==
  In1 : Inport
  In2 : Inport  Port=2
  PWR : Inport  Port=3
  Out1 : Outport
  Out2 : Outport  Port=2
  wires:
  In1/1 -> Out1/1
  In2/1 -> Out2/1

== system large_model/A664_Part7_Switch1B/Switch Hardware ==
  In1 : Inport
  In2 : Inport  Port=2
  PWR : Inport  Port=3
  Out1 : Outport
  Out2 : Outport  Port=2
  wires:
  In1/1 -> Out1/1
  In2/1 -> Out2/1

== system large_model/A664_Part7_Switch2A/Switch Hardware ==
  In1 : Inport
  In2 : Inport  Port=2
  PWR : Inport  Port=3
  Out1 : Outport
  Out2 : Outport  Port=2
  wires:
  In1/1 -> Out1/1
  In2/1 -> Out2/1

== system large_model/A664_Part7_Switch2B/Switch Hardware ==
  In1 : Inport
  In2 : Inport  Port=2
  PWR : Inport  Port=3
  Out1 : Outport
  Out2 : Outport  Port=2
  wires:
  In1/1 -> Out1/1
  In2/1 -> Out2/1

== system large_model/GPM_IO_1/IO_Card ==
  RDC4002_1 : Inport
  RDC4002_2 : Inport  Port=2
  RDC4002_3 : Inport  Port=3
  RDC4002_4 : Inport  Port=4
  IRS : Inport  Port=5
  ADS1 : Inport  Port=6
  ADS2 : Inport  Port=7
  ADS3 : Inport  Port=8
  ADS4 : Inport  Port=9
  ADS5 : Inport  Port=10
  ADS6 : Inport  Port=11
  ADS7 : Inport  Port=12
  ADS8 : Inport  Port=13
  PWR_In : Inport  Port=14
  Bus Creator : BusCreator  Inputs=13
  IO Card Hardware : Subsystem
  A429_Out1 : Outport
  wires:
  PWR_In/1 -> IO Card Hardware/2(PWR)
  IO Card Hardware/1(Out) -> A429_Out1/1
  Bus Creator/1 -> IO Card Hardware/1(In)  "io_card"
  RDC4002_1/1 -> Bus Creator/1
  RDC4002_2/1 -> Bus Creator/2
  RDC4002_3/1 -> Bus Creator/3
  RDC4002_4/1 -> Bus Creator/4
  IRS/1 -> Bus Creator/5
  ADS1/1 -> Bus Creator/6
  ADS2/1 -> Bus Creator/7
  ADS3/1 -> Bus Creator/8
  ADS4/1 -> Bus Creator/9
  ADS5/1 -> Bus Creator/10
  ADS6/1 -> Bus Creator/11
  ADS7/1 -> Bus Creator/12
  ADS8/1 -> Bus Creator/13

== system large_model/GPM_IO_1/Integrated_GPM0 ==
  From_IO_Card : Inport
  PWR_In : Inport  Port=2
  From_Switch_ChA : Inport  Port=3
  From_Switch_ChB : Inport  Port=4
  End_System : Subsystem
  GPM : Subsystem
  To_Switch_ChA : Outport
  To_Switch_ChB : Outport  Port=2
  wires:
  GPM/1(To_ES) -> End_System/1(From_GPM)
  GPM/2(PWR_Out) -> End_System/4(PWR)
  From_IO_Card/1 -> GPM/1(From_IO)
  From_Switch_ChA/1 -> End_System/2(From_Switch_ChA)
  End_System/2(To_Switch_ChA) -> To_Switch_ChA/1
  From_Switch_ChB/1 -> End_System/3(From_Switch_ChB)
  End_System/3(To_Switch_ChB) -> To_Switch_ChB/1
  PWR_In/1 -> GPM/2(PWR_In)

== system large_model/GPM_IO_2/IO_Card ==
  RDC4002_1 : Inport
  RDC4002_2 : Inport  Port=2
  RDC4002_3 : Inport  Port=3
  RDC4002_4 : Inport  Port=4
  IRS : Inport  Port=5
  ADS1 : Inport  Port=6
  ADS2 : Inport  Port=7
  ADS3 : Inport  Port=8
  ADS4 : Inport  Port=9
  ADS5 : Inport  Port=10
  ADS6 : Inport  Port=11
  ADS7 : Inport  Port=12
  ADS8 : Inport  Port=13
  PWR_In : Inport  Port=14
  Bus Creator : BusCreator  Inputs=13
  IO Card Hardware : Subsystem
  A429_Out1 : Outport
  wires:
  PWR_In/1 -> IO Card Hardware/2(PWR)
  IO Card Hardware/1(Out) -> A429_Out1/1
  Bus Creator/1 -> IO Card Hardware/1(In)  "io_card"
  RDC4002_1/1 -> Bus Creator/1
  RDC4002_2/1 -> Bus Creator/2
  RDC4002_3/1 -> Bus Creator/3
  RDC4002_4/1 -> Bus Creator/4
  IRS/1 -> Bus Creator/5
  ADS1/1 -> Bus Creator/6
  ADS2/1 -> Bus Creator/7
  ADS3/1 -> Bus Creator/8
  ADS4/1 -> Bus Creator/9
  ADS5/1 -> Bus Creator/10
  ADS6/1 -> Bus Creator/11
  ADS7/1 -> Bus Creator/12
  ADS8/1 -> Bus Creator/13

== system large_model/GPM_IO_2/Integrated_GPM0 ==
  From_IO_Card : Inport
  PWR_In : Inport  Port=2
  From_Switch_ChA : Inport  Port=3
  From_Switch_ChB : Inport  Port=4
  End_System : Subsystem
  GPM : Subsystem
  To_Switch_ChA : Outport
  To_Switch_ChB : Outport  Port=2
  wires:
  GPM/1(To_ES) -> End_System/1(From_GPM)
  GPM/2(PWR_Out) -> End_System/4(PWR)
  From_IO_Card/1 -> GPM/1(From_IO)
  From_Switch_ChA/1 -> End_System/2(From_Switch_ChA)
  End_System/2(To_Switch_ChA) -> To_Switch_ChA/1
  From_Switch_ChB/1 -> End_System/3(From_Switch_ChB)
  End_System/3(To_Switch_ChB) -> To_Switch_ChB/1
  PWR_In/1 -> GPM/2(PWR_In)

== system large_model/GPM_IO_3/IO_Card ==
  RDC4002_1 : Inport
  RDC4002_2 : Inport  Port=2
  RDC4002_3 : Inport  Port=3
  RDC4002_4 : Inport  Port=4
  IRS : Inport  Port=5
  ADS1 : Inport  Port=6
  ADS2 : Inport  Port=7
  ADS3 : Inport  Port=8
  ADS4 : Inport  Port=9
  ADS5 : Inport  Port=10
  ADS6 : Inport  Port=11
  ADS7 : Inport  Port=12
  ADS8 : Inport  Port=13
  PWR_In : Inport  Port=14
  Bus Creator : BusCreator  Inputs=13
  IO Card Hardware : Subsystem
  A429_Out1 : Outport
  wires:
  PWR_In/1 -> IO Card Hardware/2(PWR)
  IO Card Hardware/1(Out) -> A429_Out1/1
  Bus Creator/1 -> IO Card Hardware/1(In)  "io_card"
  RDC4002_1/1 -> Bus Creator/1
  RDC4002_2/1 -> Bus Creator/2
  RDC4002_3/1 -> Bus Creator/3
  RDC4002_4/1 -> Bus Creator/4
  IRS/1 -> Bus Creator/5
  ADS1/1 -> Bus Creator/6
  ADS2/1 -> Bus Creator/7
  ADS3/1 -> Bus Creator/8
  ADS4/1 -> Bus Creator/9
  ADS5/1 -> Bus Creator/10
  ADS6/1 -> Bus Creator/11
  ADS7/1 -> Bus Creator/12
  ADS8/1 -> Bus Creator/13

== system large_model/GPM_IO_3/Integrated_GPM0 ==
  From_IO_Card : Inport
  PWR_In : Inport  Port=2
  From_Switch_ChA : Inport  Port=3
  From_Switch_ChB : Inport  Port=4
  End_System : Subsystem
  GPM : Subsystem
  To_Switch_ChA : Outport
  To_Switch_ChB : Outport  Port=2
  wires:
  GPM/1(To_ES) -> End_System/1(From_GPM)
  GPM/2(PWR_Out) -> End_System/4(PWR)
  From_IO_Card/1 -> GPM/1(From_IO)
  From_Switch_ChA/1 -> End_System/2(From_Switch_ChA)
  End_System/2(To_Switch_ChA) -> To_Switch_ChA/1
  From_Switch_ChB/1 -> End_System/3(From_Switch_ChB)
  End_System/3(To_Switch_ChB) -> To_Switch_ChB/1
  PWR_In/1 -> GPM/2(PWR_In)

== system large_model/GPM_IO_4/IO_Card ==
  RDC4002_1 : Inport
  RDC4002_2 : Inport  Port=2
  RDC4002_3 : Inport  Port=3
  RDC4002_4 : Inport  Port=4
  IRS : Inport  Port=5
  ADS1 : Inport  Port=6
  ADS2 : Inport  Port=7
  ADS3 : Inport  Port=8
  ADS4 : Inport  Port=9
  ADS5 : Inport  Port=10
  ADS6 : Inport  Port=11
  ADS7 : Inport  Port=12
  ADS8 : Inport  Port=13
  PWR_In : Inport  Port=14
  Bus Creator : BusCreator  Inputs=13
  IO Card Hardware : Subsystem
  A429_Out1 : Outport
  wires:
  PWR_In/1 -> IO Card Hardware/2(PWR)
  IO Card Hardware/1(Out) -> A429_Out1/1
  Bus Creator/1 -> IO Card Hardware/1(In)  "io_card"
  RDC4002_1/1 -> Bus Creator/1
  RDC4002_2/1 -> Bus Creator/2
  RDC4002_3/1 -> Bus Creator/3
  RDC4002_4/1 -> Bus Creator/4
  IRS/1 -> Bus Creator/5
  ADS1/1 -> Bus Creator/6
  ADS2/1 -> Bus Creator/7
  ADS3/1 -> Bus Creator/8
  ADS4/1 -> Bus Creator/9
  ADS5/1 -> Bus Creator/10
  ADS6/1 -> Bus Creator/11
  ADS7/1 -> Bus Creator/12
  ADS8/1 -> Bus Creator/13

== system large_model/GPM_IO_4/Integrated_GPM0 ==
  From_IO_Card : Inport
  PWR_In : Inport  Port=2
  From_Switch_ChA : Inport  Port=3
  From_Switch_ChB : Inport  Port=4
  End_System : Subsystem
  GPM : Subsystem
  To_Switch_ChA : Outport
  To_Switch_ChB : Outport  Port=2
  wires:
  GPM/1(To_ES) -> End_System/1(From_GPM)
  GPM/2(PWR_Out) -> End_System/4(PWR)
  From_IO_Card/1 -> GPM/1(From_IO)
  From_Switch_ChA/1 -> End_System/2(From_Switch_ChA)
  End_System/2(To_Switch_ChA) -> To_Switch_ChA/1
  From_Switch_ChB/1 -> End_System/3(From_Switch_ChB)
  End_System/3(To_Switch_ChB) -> To_Switch_ChB/1
  PWR_In/1 -> GPM/2(PWR_In)

== system large_model/Integrated_GPM1_1/End_System ==
  From_GPM : Inport
  From_Switch_ChA : Inport  Port=2
  From_Switch_ChB : Inport  Port=3
  PWR : Inport  Port=4
  Bus Creator : BusCreator  Inputs=2
  ES Hardware : Subsystem
  To_GPM : Outport
  To_Switch_ChA : Outport  Port=2
  To_Switch_ChB : Outport  Port=3
  wires:
  From_Switch_ChA/1 -> Bus Creator/1
  From_Switch_ChB/1 -> Bus Creator/2
  ES Hardware/1(Out1) -> To_Switch_ChA/1, To_Switch_ChB/1
  ES Hardware/2(Out2) -> To_GPM/1
  PWR/1 -> ES Hardware/3(PWR)
  Bus Creator/1 -> ES Hardware/2(In2)
  From_GPM/1 -> ES Hardware/1(In1)
